simulink model "l8_ver118_07"  (Simulink 7.9) (continued)
== system l8_ver118_07/s1 == (continued)
      mask help:
      | Standard snap block, but with the following optional extras;
      |  1) Optionally delay the fabric trigger by a user-specified number of valids.
      |  2) Capture continuously after a trigger until a stop pulse is received. Data is captured into BRAM continuously, with old data being overwritten until a stop command is received. Then stop capturing when memory is filled. This enables you to get some data before and some data after the stop pulse. Use the 'tr_en_cnt' register to determine where you are in your vector. 'Addr' register's MSb now indicates 'busy capturing'.
      |  3) Optionally capture a value on an input port to a register at the same time as the first data sample.
      |  4) Implement counters using DSP48Es to save resources.
      mask init: same as l8_ver118_07/adcsnap0
      mask StyleString: popup(bram|dram),popup(1|2|3|4),popup(200|250|266|300|333),edit,popup(8|16|32|64|128),checkbox,checkbox,checkbox,checkbox
      mask CallbackString: snapshot_callback();||||||||
  p1im_firstcic : Subsystem  AncestorBlock=casper_library_scopes/snapshot  mask(snapshot){storage=bram, dram_dimm=1, dram_clock=200, nsamples=12, data_width=32, offset=off, circap=off, value=off, use_dsp48=on}
      mask prompts: storage "Storage medium"; dram_dimm "DRAM dimm"; dram_clock "DRAM clock rate"; nsamples "Number of Samples (2^?)"; data_width "Data width"; offset "Start delay support"; circap "Circular capture support"; value "Extra value capture support"; use_dsp48 "Use DSP48s to implement counters"
      mask help:
      | Standard snap block, but with the following optional extras;
      |  1) Optionally delay the fabric trigger by a user-specified number of valids.
      |  2) Capture continuously after a trigger until a stop pulse is received. Data is captured into BRAM continuously, with old data being overwritten until a stop command is received. Then stop capturing when memory is filled. This enables you to get some data before and some data after the stop pulse. Use the 'tr_en_cnt' register to determine where you are in your vector. 'Addr' register's MSb now indicates 'busy capturing'.
      |  3) Optionally capture a value on an input port to a register at the same time as the first data sample.
      |  4) Implement counters using DSP48Es to save resources.
      mask init: same as l8_ver118_07/adcsnap0
      mask StyleString: popup(bram|dram),popup(1|2|3|4),popup(200|250|266|300|333),edit,popup(8|16|32|64|128),checkbox,checkbox,checkbox,checkbox
      mask CallbackString: snapshot_callback();||||||||
  p1im_firstcic_full : Subsystem  AncestorBlock=casper_library_scopes/snapshot  mask(snapshot){storage=bram, dram_dimm=1, dram_clock=200, nsamples=12, data_width=64, offset=off, circap=off, value=off, use_dsp48=on}
      mask prompts: storage "Storage medium"; dram_dimm "DRAM dimm"; dram_clock "DRAM clock rate"; nsamples "Number of Samples (2^?)"; data_width "Data width"; offset "Start delay support"; circap "Circular capture support"; value "Extra value capture support"; use_dsp48 "Use DSP48s to implement counters"
      mask help:
      | Standard snap block, but with the following optional extras;
      |  1) Optionally delay the fabric trigger by a user-specified number of valids.
      |  2) Capture continuously after a trigger until a stop pulse is received. Data is captured into BRAM continuously, with old data being overwritten until a stop command is received. Then stop capturing when memory is filled. This enables you to get some data before and some data after the stop pulse. Use the 'tr_en_cnt' register to determine where you are in your vector. 'Addr' register's MSb now indicates 'busy capturing'.
      |  3) Optionally capture a value on an input port to a register at the same time as the first data sample.
      |  4) Implement counters using DSP48Es to save resources.
      mask init: same as l8_ver118_07/adcsnap0
      mask StyleString: popup(bram|dram),popup(1|2|3|4),popup(200|250|266|300|333),edit,popup(8|16|32|64|128),checkbox,checkbox,checkbox,checkbox
      mask CallbackString: snapshot_callback();||||||||
  p1im_halfband : Subsystem  AncestorBlock=casper_library_scopes/snapshot  mask(snapshot){storage=bram, dram_dimm=1, dram_clock=200, nsamples=12, data_width=32, offset=off, circap=off, value=off, use_dsp48=on}
      mask prompts: storage "Storage medium"; dram_dimm "DRAM dimm"; dram_clock "DRAM clock rate"; nsamples "Number of Samples (2^?)"; data_width "Data width"; offset "Start delay support"; circap "Circular capture support"; value "Extra value capture support"; use_dsp48 "Use DSP48s to implement counters"
      mask help:
      | Standard snap block, but with the following optional extras;
      |  1) Optionally delay the fabric trigger by a user-specified number of valids.
      |  2) Capture continuously after a trigger until a stop pulse is received. Data is captured into BRAM continuously, with old data being overwritten until a stop command is received. Then stop capturing when memory is filled. This enables you to get some data before and some data after the stop pulse. Use the 'tr_en_cnt' register to determine where you are in your vector. 'Addr' register's MSb now indicates 'busy capturing'.
      |  3) Optionally capture a value on an input port to a register at the same time as the first data sample.
      |  4) Implement counters using DSP48Es to save resources.
      mask init: same as l8_ver118_07/adcsnap0
      mask StyleString: popup(bram|dram),popup(1|2|3|4),popup(200|250|266|300|333),edit,popup(8|16|32|64|128),checkbox,checkbox,checkbox,checkbox
      mask CallbackString: snapshot_callback();||||||||
  p1re_dr16 : Subsystem  AncestorBlock=casper_library_scopes/snapshot  mask(snapshot){storage=bram, dram_dimm=1, dram_clock=200, nsamples=12, data_width=64, offset=off, circap=off, value=off, use_dsp48=on}
      mask prompts: storage "Storage medium"; dram_dimm "DRAM dimm"; dram_clock "DRAM clock rate"; nsamples "Number of Samples (2^?)"; data_width "Data width"; offset "Start delay support"; circap "Circular capture support"; value "Extra value capture support"; use_dsp48 "Use DSP48s to implement counters"
      mask help:
      | Standard snap block, but with the following optional extras;
      |  1) Optionally delay the fabric trigger by a user-specified number of valids.
      |  2) Capture continuously after a trigger until a stop pulse is received. Data is captured into BRAM continuously, with old data being overwritten until a stop command is received. Then stop capturing when memory is filled. This enables you to get some data before and some data after the stop pulse. Use the 'tr_en_cnt' register to determine where you are in your vector. 'Addr' register's MSb now indicates 'busy capturing'.
      |  3) Optionally capture a value on an input port to a register at the same time as the first data sample.
      |  4) Implement counters using DSP48Es to save resources.
      mask init: same as l8_ver118_07/adcsnap0
      mask StyleString: popup(bram|dram),popup(1|2|3|4),popup(200|250|266|300|333),edit,popup(8|16|32|64|128),checkbox,checkbox,checkbox,checkbox
      mask CallbackString: snapshot_callback();||||||||
  p1re_firstcic : Subsystem  AncestorBlock=casper_library_scopes/snapshot  mask(snapshot){storage=bram, dram_dimm=1, dram_clock=200, nsamples=12, data_width=32, offset=off, circap=off, value=off, use_dsp48=on}
      mask prompts: storage "Storage medium"; dram_dimm "DRAM dimm"; dram_clock "DRAM clock rate"; nsamples "Number of Samples (2^?)"; data_width "Data width"; offset "Start delay support"; circap "Circular capture support"; value "Extra value capture support"; use_dsp48 "Use DSP48s to implement counters"
      mask help:
      | Standard snap block, but with the following optional extras;
      |  1) Optionally delay the fabric trigger by a user-specified number of valids.
      |  2) Capture continuously after a trigger until a stop pulse is received. Data is captured into BRAM continuously, with old data being overwritten until a stop command is received. Then stop capturing when memory is filled. This enables you to get some data before and some data after the stop pulse. Use the 'tr_en_cnt' register to determine where you are in your vector. 'Addr' register's MSb now indicates 'busy capturing'.
      |  3) Optionally capture a value on an input port to a register at the same time as the first data sample.
      |  4) Implement counters using DSP48Es to save resources.
      mask init: same as l8_ver118_07/adcsnap0
      mask StyleString: popup(bram|dram),popup(1|2|3|4),popup(200|250|266|300|333),edit,popup(8|16|32|64|128),checkbox,checkbox,checkbox,checkbox
      mask CallbackString: snapshot_callback();||||||||
  p1re_firstcic_full : Subsystem  AncestorBlock=casper_library_scopes/snapshot  mask(snapshot){storage=bram, dram_dimm=1, dram_clock=200, nsamples=12, data_width=64, offset=off, circap=off, value=off, use_dsp48=on}
      mask prompts: storage "Storage medium"; dram_dimm "DRAM dimm"; dram_clock "DRAM clock rate"; nsamples "Number of Samples (2^?)"; data_width "Data width"; offset "Start delay support"; circap "Circular capture support"; value "Extra value capture support"; use_dsp48 "Use DSP48s to implement counters"
      mask help:
      | Standard snap block, but with the following optional extras;
      |  1) Optionally delay the fabric trigger by a user-specified number of valids.
      |  2) Capture continuously after a trigger until a stop pulse is received. Data is captured into BRAM continuously, with old data being overwritten until a stop command is received. Then stop capturing when memory is filled. This enables you to get some data before and some data after the stop pulse. Use the 'tr_en_cnt' register to determine where you are in your vector. 'Addr' register's MSb now indicates 'busy capturing'.
      |  3) Optionally capture a value on an input port to a register at the same time as the first data sample.
      |  4) Implement counters using DSP48Es to save resources.
      mask init: same as l8_ver118_07/adcsnap0
      mask StyleString: popup(bram|dram),popup(1|2|3|4),popup(200|250|266|300|333),edit,popup(8|16|32|64|128),checkbox,checkbox,checkbox,checkbox
      mask CallbackString: snapshot_callback();||||||||
  p1re_halfband : Subsystem  AncestorBlock=casper_library_scopes/snapshot  mask(snapshot){storage=bram, dram_dimm=1, dram_clock=200, nsamples=12, data_width=32, offset=off, circap=off, value=off, use_dsp48=on}
      mask prompts: storage "Storage medium"; dram_dimm "DRAM dimm"; dram_clock "DRAM clock rate"; nsamples "Number of Samples (2^?)"; data_width "Data width"; offset "Start delay support"; circap "Circular capture support"; value "Extra value capture support"; use_dsp48 "Use DSP48s to implement counters"
      mask help:
      | Standard snap block, but with the following optional extras;
      |  1) Optionally delay the fabric trigger by a user-specified number of valids.
      |  2) Capture continuously after a trigger until a stop pulse is received. Data is captured into BRAM continuously, with old data being overwritten until a stop command is received. Then stop capturing when memory is filled. This enables you to get some data before and some data after the stop pulse. Use the 'tr_en_cnt' register to determine where you are in your vector. 'Addr' register's MSb now indicates 'busy capturing'.
      |  3) Optionally capture a value on an input port to a register at the same time as the first data sample.
      |  4) Implement counters using DSP48Es to save resources.
      mask init: same as l8_ver118_07/adcsnap0
      mask StyleString: popup(bram|dram),popup(1|2|3|4),popup(200|250|266|300|333),edit,popup(8|16|32|64|128),checkbox,checkbox,checkbox,checkbox
      mask CallbackString: snapshot_callback();||||||||
  p2im_dr16 : Subsystem  AncestorBlock=casper_library_scopes/snapshot  mask(snapshot){storage=bram, dram_dimm=1, dram_clock=200, nsamples=12, data_width=64, offset=off, circap=off, value=off, use_dsp48=on}
      mask prompts: storage "Storage medium"; dram_dimm "DRAM dimm"; dram_clock "DRAM clock rate"; nsamples "Number of Samples (2^?)"; data_width "Data width"; offset "Start delay support"; circap "Circular capture support"; value "Extra value capture support"; use_dsp48 "Use DSP48s to implement counters"
      mask help:
      | Standard snap block, but with the following optional extras;
      |  1) Optionally delay the fabric trigger by a user-specified number of valids.
      |  2) Capture continuously after a trigger until a stop pulse is received. Data is captured into BRAM continuously, with old data being overwritten until a stop command is received. Then stop capturing when memory is filled. This enables you to get some data before and some data after the stop pulse. Use the 'tr_en_cnt' register to determine where you are in your vector. 'Addr' register's MSb now indicates 'busy capturing'.
      |  3) Optionally capture a value on an input port to a register at the same time as the first data sample.
      |  4) Implement counters using DSP48Es to save resources.
      mask init: same as l8_ver118_07/adcsnap0
      mask StyleString: popup(bram|dram),popup(1|2|3|4),popup(200|250|266|300|333),edit,popup(8|16|32|64|128),checkbox,checkbox,checkbox,checkbox
      mask CallbackString: snapshot_callback();||||||||
  p2im_firstcic : Subsystem  AncestorBlock=casper_library_scopes/snapshot  mask(snapshot){storage=bram, dram_dimm=1, dram_clock=200, nsamples=12, data_width=32, offset=off, circap=off, value=off, use_dsp48=on}
      mask prompts: storage "Storage medium"; dram_dimm "DRAM dimm"; dram_clock "DRAM clock rate"; nsamples "Number of Samples (2^?)"; data_width "Data width"; offset "Start delay support"; circap "Circular capture support"; value "Extra value capture support"; use_dsp48 "Use DSP48s to implement counters"
      mask help:
      | Standard snap block, but with the following optional extras;
      |  1) Optionally delay the fabric trigger by a user-specified number of valids.
      |  2) Capture continuously after a trigger until a stop pulse is received. Data is captured into BRAM continuously, with old data being overwritten until a stop command is received. Then stop capturing when memory is filled. This enables you to get some data before and some data after the stop pulse. Use the 'tr_en_cnt' register to determine where you are in your vector. 'Addr' register's MSb now indicates 'busy capturing'.
      |  3) Optionally capture a value on an input port to a register at the same time as the first data sample.
      |  4) Implement counters using DSP48Es to save resources.
      mask init: same as l8_ver118_07/adcsnap0
      mask StyleString: popup(bram|dram),popup(1|2|3|4),popup(200|250|266|300|333),edit,popup(8|16|32|64|128),checkbox,checkbox,checkbox,checkbox
      mask CallbackString: snapshot_callback();||||||||
  p2im_firstcic_full : Subsystem  AncestorBlock=casper_library_scopes/snapshot  mask(snapshot){storage=bram, dram_dimm=1, dram_clock=200, nsamples=12, data_width=64, offset=off, circap=off, value=off, use_dsp48=on}
      mask prompts: storage "Storage medium"; dram_dimm "DRAM dimm"; dram_clock "DRAM clock rate"; nsamples "Number of Samples (2^?)"; data_width "Data width"; offset "Start delay support"; circap "Circular capture support"; value "Extra value capture support"; use_dsp48 "Use DSP48s to implement counters"
      mask help:
      | Standard snap block, but with the following optional extras;
      |  1) Optionally delay the fabric trigger by a user-specified number of valids.
      |  2) Capture continuously after a trigger until a stop pulse is received. Data is captured into BRAM continuously, with old data being overwritten until a stop command is received. Then stop capturing when memory is filled. This enables you to get some data before and some data after the stop pulse. Use the 'tr_en_cnt' register to determine where you are in your vector. 'Addr' register's MSb now indicates 'busy capturing'.
      |  3) Optionally capture a value on an input port to a register at the same time as the first data sample.
      |  4) Implement counters using DSP48Es to save resources.
      mask init: same as l8_ver118_07/adcsnap0
      mask StyleString: popup(bram|dram),popup(1|2|3|4),popup(200|250|266|300|333),edit,popup(8|16|32|64|128),checkbox,checkbox,checkbox,checkbox
      mask CallbackString: snapshot_callback();||||||||
  p2im_halfband : Subsystem  AncestorBlock=casper_library_scopes/snapshot  mask(snapshot){storage=bram, dram_dimm=1, dram_clock=200, nsamples=12, data_width=32, offset=off, circap=off, value=off, use_dsp48=on}
      mask prompts: storage "Storage medium"; dram_dimm "DRAM dimm"; dram_clock "DRAM clock rate"; nsamples "Number of Samples (2^?)"; data_width "Data width"; offset "Start delay support"; circap "Circular capture support"; value "Extra value capture support"; use_dsp48 "Use DSP48s to implement counters"
      mask help:
      | Standard snap block, but with the following optional extras;
      |  1) Optionally delay the fabric trigger by a user-specified number of valids.
      |  2) Capture continuously after a trigger until a stop pulse is received. Data is captured into BRAM continuously, with old data being overwritten until a stop command is received. Then stop capturing when memory is filled. This enables you to get some data before and some data after the stop pulse. Use the 'tr_en_cnt' register to determine where you are in your vector. 'Addr' register's MSb now indicates 'busy capturing'.
      |  3) Optionally capture a value on an input port to a register at the same time as the first data sample.
      |  4) Implement counters using DSP48Es to save resources.
      mask init: same as l8_ver118_07/adcsnap0
      mask StyleString: popup(bram|dram),popup(1|2|3|4),popup(200|250|266|300|333),edit,popup(8|16|32|64|128),checkbox,checkbox,checkbox,checkbox
      mask CallbackString: snapshot_callback();||||||||
  p2re_dr16 : Subsystem  AncestorBlock=casper_library_scopes/snapshot  mask(snapshot){storage=bram, dram_dimm=1, dram_clock=200, nsamples=12, data_width=64, offset=off, circap=off, value=off, use_dsp48=on}
      mask prompts: storage "Storage medium"; dram_dimm "DRAM dimm"; dram_clock "DRAM clock rate"; nsamples "Number of Samples (2^?)"; data_width "Data width"; offset "Start delay support"; circap "Circular capture support"; value "Extra value capture support"; use_dsp48 "Use DSP48s to implement counters"
      mask help:
      | Standard snap block, but with the following optional extras;
      |  1) Optionally delay the fabric trigger by a user-specified number of valids.
      |  2) Capture continuously after a trigger until a stop pulse is received. Data is captured into BRAM continuously, with old data being overwritten until a stop command is received. Then stop capturing when memory is filled. This enables you to get some data before and some data after the stop pulse. Use the 'tr_en_cnt' register to determine where you are in your vector. 'Addr' register's MSb now indicates 'busy capturing'.
      |  3) Optionally capture a value on an input port to a register at the same time as the first data sample.
      |  4) Implement counters using DSP48Es to save resources.
      mask init: same as l8_ver118_07/adcsnap0
      mask StyleString: popup(bram|dram),popup(1|2|3|4),popup(200|250|266|300|333),edit,popup(8|16|32|64|128),checkbox,checkbox,checkbox,checkbox
      mask CallbackString: snapshot_callback();||||||||
  p2re_firstcic : Subsystem  AncestorBlock=casper_library_scopes/snapshot  mask(snapshot){storage=bram, dram_dimm=1, dram_clock=200, nsamples=12, data_width=32, offset=off, circap=off, value=off, use_dsp48=on}
      mask prompts: storage "Storage medium"; dram_dimm "DRAM dimm"; dram_clock "DRAM clock rate"; nsamples "Number of Samples (2^?)"; data_width "Data width"; offset "Start delay support"; circap "Circular capture support"; value "Extra value capture support"; use_dsp48 "Use DSP48s to implement counters"
      mask help:
      | Standard snap block, but with the following optional extras;
      |  1) Optionally delay the fabric trigger by a user-specified number of valids.
      |  2) Capture continuously after a trigger until a stop pulse is received. Data is captured into BRAM continuously, with old data being overwritten until a stop command is received. Then stop capturing when memory is filled. This enables you to get some data before and some data after the stop pulse. Use the 'tr_en_cnt' register to determine where you are in your vector. 'Addr' register's MSb now indicates 'busy capturing'.
      |  3) Optionally capture a value on an input port to a register at the same time as the first data sample.
      |  4) Implement counters using DSP48Es to save resources.
      mask init: same as l8_ver118_07/adcsnap0
      mask StyleString: popup(bram|dram),popup(1|2|3|4),popup(200|250|266|300|333),edit,popup(8|16|32|64|128),checkbox,checkbox,checkbox,checkbox
      mask CallbackString: snapshot_callback();||||||||
  p2re_firstcic_full : Subsystem  AncestorBlock=casper_library_scopes/snapshot  mask(snapshot){storage=bram, dram_dimm=1, dram_clock=200, nsamples=12, data_width=64, offset=off, circap=off, value=off, use_dsp48=on}
      mask prompts: storage "Storage medium"; dram_dimm "DRAM dimm"; dram_clock "DRAM clock rate"; nsamples "Number of Samples (2^?)"; data_width "Data width"; offset "Start delay support"; circap "Circular capture support"; value "Extra value capture support"; use_dsp48 "Use DSP48s to implement counters"
      mask help:
      | Standard snap block, but with the following optional extras;
      |  1) Optionally delay the fabric trigger by a user-specified number of valids.
      |  2) Capture continuously after a trigger until a stop pulse is received. Data is captured into BRAM continuously, with old data being overwritten until a stop command is received. Then stop capturing when memory is filled. This enables you to get some data before and some data after the stop pulse. Use the 'tr_en_cnt' register to determine where you are in your vector. 'Addr' register's MSb now indicates 'busy capturing'.
      |  3) Optionally capture a value on an input port to a register at the same time as the first data sample.
      |  4) Implement counters using DSP48Es to save resources.
      mask init: same as l8_ver118_07/adcsnap0
      mask StyleString: popup(bram|dram),popup(1|2|3|4),popup(200|250|266|300|333),edit,popup(8|16|32|64|128),checkbox,checkbox,checkbox,checkbox
      mask CallbackString: snapshot_callback();||||||||
  p2re_halfband : Subsystem  AncestorBlock=casper_library_scopes/snapshot  mask(snapshot){storage=bram, dram_dimm=1, dram_clock=200, nsamples=12, data_width=32, offset=off, circap=off, value=off, use_dsp48=on}
      mask prompts: storage "Storage medium"; dram_dimm "DRAM dimm"; dram_clock "DRAM clock rate"; nsamples "Number of Samples (2^?)"; data_width "Data width"; offset "Start delay support"; circap "Circular capture support"; value "Extra value capture support"; use_dsp48 "Use DSP48s to implement counters"
      mask help:
      | Standard snap block, but with the following optional extras;
      |  1) Optionally delay the fabric trigger by a user-specified number of valids.
      |  2) Capture continuously after a trigger until a stop pulse is received. Data is captured into BRAM continuously, with old data being overwritten until a stop command is received. Then stop capturing when memory is filled. This enables you to get some data before and some data after the stop pulse. Use the 'tr_en_cnt' register to determine where you are in your vector. 'Addr' register's MSb now indicates 'busy capturing'.
      |  3) Optionally capture a value on an input port to a register at the same time as the first data sample.
      |  4) Implement counters using DSP48Es to save resources.
      mask init: same as l8_ver118_07/adcsnap0
      mask StyleString: popup(bram|dram),popup(1|2|3|4),popup(200|250|266|300|333),edit,popup(8|16|32|64|128),checkbox,checkbox,checkbox,checkbox
      mask CallbackString: snapshot_callback();||||||||
  quant : Subsystem
  pol1_re : Outport
  pol1_im : Outport  Port=2
  pol2_re : Outport  Port=3
  pol2_im : Outport  Port=4
  sync : Outport  Port=5
  wires:
  pol1_in0/1 -> [pol1_0]
  pol1_in1/1 -> [pol1_1]
  pol1_in2/1 -> [pol1_2]
  pol1_in3/1 -> [pol1_3]
  pol1_in4/1 -> [pol1_4]
  pol1_in5/1 -> [pol1_5]
  pol1_in6/1 -> [pol1_6]
  pol1_in7/1 -> [pol1_7]
  pol2_in0/1 -> [pol2_0]
  pol2_in1/1 -> [pol2_1]
  pol2_in2/1 -> [pol2_2]
  pol2_in3/1 -> [pol2_3]
  pol2_in4/1 -> [pol2_4]
  pol2_in5/1 -> [pol2_5]
  pol2_in6/1 -> [pol2_6]
  pol2_in7/1 -> [pol2_7]
  [mixer_addr] -> lo_2/1(addr)
  [mixer_addr] -> lo_4/1(addr)
  [mixer_addr] -> lo_6/1(addr)
  lo_8/1(pol1_im) -> [pol1_imag_8]
  lo_8/2(pol2_im) -> [pol2_imag_8]
  lo_8/3(pol1_re) -> [pol1_real_8]
  lo_8/4(pol2_re) -> [pol2_real_8]
  [mixer_addr] -> lo_8/1(addr)
  [pol2_8] -> lo_8/3(pol2)
  [pol1_8] -> lo_8/2(pol1)
  [mixer_addr] -> lo_0/1(addr)
  [pol1_0] -> lo_0/2(pol1)
  [pol2_0] -> lo_0/3(pol2)
  lo_0/1(pol1_im) -> [pol1_imag_0]
  lo_0/2(pol2_im) -> [pol2_imag_0]
  lo_0/3(pol1_re) -> [pol1_real_0]
  lo_0/4(pol2_re) -> [pol2_real_0]
  [pol1_2] -> lo_2/2(pol1)
  [pol2_2] -> lo_2/3(pol2)
  [pol1_4] -> lo_4/2(pol1)
  [pol2_4] -> lo_4/3(pol2)
  [pol1_6] -> lo_6/2(pol1)
  [pol2_6] -> lo_6/3(pol2)
  lo_6/1(pol1_im) -> [pol1_imag_6]
  lo_6/2(pol2_im) -> [pol2_imag_6]
  lo_6/3(pol1_re) -> [pol1_real_6]
  lo_6/4(pol2_re) -> [pol2_real_6]
  [mixer_addr] -> lo_10/1(addr)
  [pol1_10] -> lo_10/2(pol1)
  [pol2_10] -> lo_10/3(pol2)
  lo_10/1(pol1_im) -> [pol1_imag_10]
  lo_10/2(pol2_im) -> [pol2_imag_10]
  lo_10/3(pol1_re) -> [pol1_real_10]
  lo_10/4(pol2_re) -> [pol2_real_10]
  [mixer_addr] -> lo_12/1(addr)
  [pol1_12] -> lo_12/2(pol1)
  [pol2_12] -> lo_12/3(pol2)
  lo_12/1(pol1_im) -> [pol1_imag_12]
  lo_12/2(pol2_im) -> [pol2_imag_12]
  lo_12/3(pol1_re) -> [pol1_real_12]
  lo_12/4(pol2_re) -> [pol2_real_12]
  [mixer_addr] -> lo_14/1(addr)
  [pol1_14] -> lo_14/2(pol1)
  [pol2_14] -> lo_14/3(pol2)
  lo_14/1(pol1_im) -> [pol1_imag_14]
  lo_14/2(pol2_im) -> [pol2_imag_14]
  lo_14/3(pol1_re) -> [pol1_real_14]
  lo_14/4(pol2_re) -> [pol2_real_14]
  lo_4/1(pol1_im) -> [pol1_imag_4]
  lo_4/2(pol2_im) -> [pol2_imag_4]
  lo_4/3(pol1_re) -> [pol1_real_4]
  lo_4/4(pol2_re) -> [pol2_real_4]
  adc_CIC_sync/1 -> [cic_sync]
  [pol1_real_quant] -> pol1_re/1
  cic_stage_dec2/2(sync_out) -> cic_stage_dec1/2(sync_in)
  cic_stage_dec2/1(out) -> cic_stage_dec1/1(in)
  Halfband0/1(out) -> Convert1/1
  [cic_sync] -> cic_stage_dec2/2(sync_in)
  Convert2/1 -> Halfband0/1(in), [pol1_real_1stcic]
  Convert1/1 -> cic_stage_dec2/1(in), [pol1_real_halfband]
  Convert3/1 -> [pol1_real]
  [pol1_imag_quant] -> pol1_im/1
  quant/1(Out1) -> [pol1_real_quant]
  quant/2(Out2) -> [pol1_imag_quant]
  [pol1_real] -> quant/1(In2)
  [pol1_imag] -> quant/2(In3)
  [pol2_real] -> quant/3(In4)
  [pol2_imag] -> quant/4(In5)
  quant/3(Out3) -> [pol2_real_quant]
  quant/4(Out4) -> [pol2_imag_quant]
  [pol2_real_quant] -> pol2_re/1
  [pol2_imag_quant] -> pol2_im/1
  pol1_in8/1 -> [pol1_8]
  pol1_in9/1 -> [pol1_9]
  pol1_in10/1 -> [pol1_10]
  pol1_in11/1 -> [pol1_11]
  pol1_in12/1 -> [pol1_12]
  pol1_in13/1 -> [pol1_13]
  pol1_in14/1 -> [pol1_14]
  pol1_in15/1 -> [pol1_15]
  pol2_in8/1 -> [pol2_8]
  pol2_in9/1 -> [pol2_9]
  pol2_in10/1 -> [pol2_10]
  pol2_in11/1 -> [pol2_11]
  pol2_in12/1 -> [pol2_12]
  pol2_in13/1 -> [pol2_13]
  pol2_in14/1 -> [pol2_14]
  pol2_in15/1 -> [pol2_15]
  [mixer_addr] -> lo_1/1(addr)
  [pol1_1] -> lo_1/2(pol1)
  [pol2_1] -> lo_1/3(pol2)
  lo_1/1(pol1_im) -> [pol1_imag_1]
  lo_1/2(pol2_im) -> [pol2_imag_1]
  lo_1/3(pol1_re) -> [pol1_real_1]
  lo_1/4(pol2_re) -> [pol2_real_1]
  [mixer_addr] -> lo_9/1(addr)
  [pol1_9] -> lo_9/2(pol1)
  [pol2_9] -> lo_9/3(pol2)
  lo_9/1(pol1_im) -> [pol1_imag_9]
  lo_9/2(pol2_im) -> [pol2_imag_9]
  lo_9/3(pol1_re) -> [pol1_real_9]
  lo_9/4(pol2_re) -> [pol2_real_9]
  [mixer_addr] -> lo_3/1(addr)
  [pol1_3] -> lo_3/2(pol1)
  [pol2_3] -> lo_3/3(pol2)
  [mixer_addr] -> lo_11/1(addr)
  [pol1_11] -> lo_11/2(pol1)
  [pol2_11] -> lo_11/3(pol2)
  lo_11/1(pol1_im) -> [pol1_imag_11]
  lo_11/2(pol2_im) -> [pol2_imag_11]
  lo_11/3(pol1_re) -> [pol1_real_11]
  lo_11/4(pol2_re) -> [pol2_real_11]
  [mixer_addr] -> lo_5/1(addr)
  [pol1_5] -> lo_5/2(pol1)
  [pol2_5] -> lo_5/3(pol2)
  lo_5/1(pol1_im) -> [pol1_imag_5]
  lo_5/2(pol2_im) -> [pol2_imag_5]
  lo_5/3(pol1_re) -> [pol1_real_5]
  lo_5/4(pol2_re) -> [pol2_real_5]
  [mixer_addr] -> lo_13/1(addr)
  [pol1_13] -> lo_13/2(pol1)
  [pol2_13] -> lo_13/3(pol2)
  lo_13/1(pol1_im) -> [pol1_imag_13]
  lo_13/2(pol2_im) -> [pol2_imag_13]
  lo_13/3(pol1_re) -> [pol1_real_13]
  lo_13/4(pol2_re) -> [pol2_real_13]
  [mixer_addr] -> lo_7/1(addr)
  [pol1_7] -> lo_7/2(pol1)
  [pol2_7] -> lo_7/3(pol2)
  lo_7/1(pol1_im) -> [pol1_imag_7]
  lo_7/2(pol2_im) -> [pol2_imag_7]
  lo_7/3(pol1_re) -> [pol1_real_7]
  lo_7/4(pol2_re) -> [pol2_real_7]
  [mixer_addr] -> lo_15/1(addr)
  [pol1_15] -> lo_15/2(pol1)
  [pol2_15] -> lo_15/3(pol2)
  lo_15/1(pol1_im) -> [pol1_imag_15]
  lo_15/2(pol2_im) -> [pol2_imag_15]
  lo_15/3(pol1_re) -> [pol1_real_15]
  lo_15/4(pol2_re) -> [pol2_real_15]
  lo_2/2(pol2_im) -> [pol2_imag_2]
  lo_2/1(pol1_im) -> [pol1_imag_2]
  lo_2/3(pol1_re) -> [pol1_real_2]
  lo_2/4(pol2_re) -> [pol2_real_2]
  lo_3/1(pol1_im) -> [pol1_imag_3]
  lo_3/2(pol2_im) -> [pol2_imag_3]
  lo_3/3(pol1_re) -> [pol1_real_3]
  lo_3/4(pol2_re) -> [pol2_real_3]
  [cic_sync] -> cic_pow2_0/17(sync_in)
  [pol1_real_0] -> cic_pow2_0/1(in1)
  [pol1_real_1] -> cic_pow2_0/2(in2)
  [pol1_real_2] -> cic_pow2_0/3(in3)
  [pol1_real_3] -> cic_pow2_0/4(in4)
  [pol1_real_4] -> cic_pow2_0/5(in5)
  [pol1_real_5] -> cic_pow2_0/6(in6)
  [pol1_real_6] -> cic_pow2_0/7(in7)
  [pol1_real_7] -> cic_pow2_0/8(in8)
  [pol1_real_8] -> cic_pow2_0/9(in9)
  [pol1_real_9] -> cic_pow2_0/10(in10)
  [pol1_real_10] -> cic_pow2_0/11(in11)
  [pol1_real_11] -> cic_pow2_0/12(in12)
  [pol1_real_12] -> cic_pow2_0/13(in13)
  [pol1_real_13] -> cic_pow2_0/14(in14)
  [pol1_real_14] -> cic_pow2_0/15(in15)
  [pol1_real_15] -> cic_pow2_0/16(in16)
  cic_stage_dec1/1(out) -> Convert3/1
  cic_stage_dec1/2(sync_out) -> sync/1
  Constant19/1 -> p1re_halfband/3(trig), p1re_halfband/2(we)
  [pol1_real_halfband] -> p1re_halfband/1(din)
  Constant1/1 -> p1re_firstcic/3(trig), p1re_firstcic/2(we)
  [pol1_real_1stcic] -> p1re_firstcic/1(din)
  Reinterpret1/1 -> Convert2/1
  Constant2/1 -> mixer_p2im/2(we), mixer_p2im/3(trig)
  bus_create/1(bus_out) -> mixer_p2im/1(din)
  Slice/1 -> bus_create/1(in1)
  Slice1/1 -> bus_create/2(in2)
  Slice2/1 -> bus_create/3(in3)
  Slice3/1 -> bus_create/4(in4)
  Slice4/1 -> bus_create/5(in5)
  Slice5/1 -> bus_create/6(in6)
  Slice6/1 -> bus_create/7(in7)
  Slice7/1 -> bus_create/8(in8)
  Slice8/1 -> bus_create/9(in9)
  Slice9/1 -> bus_create/10(in10)
  Slice10/1 -> bus_create/11(in11)
  Slice11/1 -> bus_create/12(in12)
  Slice12/1 -> bus_create/13(in13)
  Slice13/1 -> bus_create/14(in14)
  Slice14/1 -> bus_create/15(in15)
  Slice15/1 -> bus_create/16(in16)
  Constant3/1 -> p1re_firstcic_full/3(trig), p1re_firstcic_full/2(we)
  [pol1_re_1stcic_full] -> Reinterpret5/1
  Reinterpret5/1 -> Convert12/1
  Convert12/1 -> p1re_firstcic_full/1(din)
  cic_stage_dec9/1(out) -> Reinterpret1/1, [pol1_re_1stcic_full]
  cic_stage_dec9/2(sync_out) -> Halfband0/2(sync)
  Constant4/1 -> p1re_dr16/3(trig), p1re_dr16/2(we)
  [pol1_re_16dr] -> Reinterpret6/1
  Reinterpret6/1 -> Convert13/1
  Convert13/1 -> p1re_dr16/1(din)
  mixer_addr/1 -> Relational/1, Delay80/1
  Delay80/1 -> [mixer_addr]
  Relational/1 -> mixer_addr/1
  mixer_cnt/1 -> Relational/2
  Constant15/1 -> mixer_cnt/1
  [pol1_imag_0] -> cic_pow2_1/1(in1)
  [pol1_imag_1] -> cic_pow2_1/2(in2)
  [pol1_imag_2] -> cic_pow2_1/3(in3)
  [pol1_imag_3] -> cic_pow2_1/4(in4)
  [pol1_imag_4] -> cic_pow2_1/5(in5)
  [pol1_imag_5] -> cic_pow2_1/6(in6)
  [pol1_imag_6] -> cic_pow2_1/7(in7)
  [pol1_imag_7] -> cic_pow2_1/8(in8)
  [pol1_imag_8] -> cic_pow2_1/9(in9)
  [pol1_imag_9] -> cic_pow2_1/10(in10)
  [pol1_imag_10] -> cic_pow2_1/11(in11)
  [pol1_imag_11] -> cic_pow2_1/12(in12)
  [pol1_imag_12] -> cic_pow2_1/13(in13)
  [pol1_imag_13] -> cic_pow2_1/14(in14)
  [pol1_imag_14] -> cic_pow2_1/15(in15)
  [pol1_imag_15] -> cic_pow2_1/16(in16)
  [cic_sync] -> cic_pow2_1/17(sync_in)
  cic_pow2_0/1(out1) -> cic_stage_dec9/1(in), [pol1_re_16dr]
  cic_pow2_0/2(sync_out) -> cic_stage_dec9/2(sync_in)
  cic_stage_dec4/2(sync_out) -> cic_stage_dec3/2(sync_in)
  cic_stage_dec4/1(out) -> cic_stage_dec3/1(in)
  Halfband1/1(out) -> Convert4/1
  [cic_sync] -> cic_stage_dec4/2(sync_in)
  Convert5/1 -> Halfband1/1(in), [pol1_imag_1stcic]
  Convert4/1 -> cic_stage_dec4/1(in), [pol1_imag_halfband]
  Convert6/1 -> [pol1_imag]
  cic_stage_dec3/1(out) -> Convert6/1
  Reinterpret2/1 -> Convert5/1
  cic_stage_dec5/1(out) -> Reinterpret2/1, [pol1_im_1stcic_full]
  cic_stage_dec5/2(sync_out) -> Halfband1/2(sync)
  cic_pow2_1/1(out1) -> cic_stage_dec5/1(in), [pol1_im_16dr]
  cic_pow2_1/2(sync_out) -> cic_stage_dec5/2(sync_in)
  Constant6/1 -> p1im_halfband/3(trig), p1im_halfband/2(we)
  [pol1_imag_halfband] -> p1im_halfband/1(din)
  Constant5/1 -> p1im_firstcic/3(trig), p1im_firstcic/2(we)
  [pol1_imag_1stcic] -> p1im_firstcic/1(din)
  Constant7/1 -> p1im_firstcic_full/3(trig), p1im_firstcic_full/2(we)
  [pol1_im_1stcic_full] -> Reinterpret3/1
  Reinterpret3/1 -> Convert7/1
  Convert7/1 -> p1im_firstcic_full/1(din)
  Constant8/1 -> p1im_dr16/3(trig), p1im_dr16/2(we)
  [pol1_im_16dr] -> Reinterpret4/1
  Reinterpret4/1 -> Convert8/1
  Convert8/1 -> p1im_dr16/1(din)
  [pol1_real_0] -> Gateway Out4/1
  [pol1_imag_0] -> Gateway Out1/1
  [pol2_real_0] -> Gateway Out2/1
  [pol2_imag_0] -> Gateway Out3/1
  Gateway Out4/1 -> Scope/1
  Gateway Out1/1 -> Scope/2
  Gateway Out2/1 -> Scope/3
  Gateway Out3/1 -> Scope/4
  cic_stage_dec7/2(sync_out) -> cic_stage_dec6/2(sync_in)
  cic_stage_dec7/1(out) -> cic_stage_dec6/1(in)
  Halfband2/1(out) -> Convert9/1
  [cic_sync] -> cic_stage_dec7/2(sync_in)
  Convert10/1 -> Halfband2/1(in), [pol2_real_1stcic]
  Convert9/1 -> cic_stage_dec7/1(in), [pol2_real_halfband]
  Convert11/1 -> [pol2_real]
  cic_stage_dec6/1(out) -> Convert11/1
  Reinterpret7/1 -> Convert10/1
  cic_stage_dec8/1(out) -> Reinterpret7/1, [pol2_re_1stcic_full]
  cic_stage_dec8/2(sync_out) -> Halfband2/2(sync)
  cic_pow2_2/1(out1) -> cic_stage_dec8/1(in), [pol2_re_16dr]
  cic_pow2_2/2(sync_out) -> cic_stage_dec8/2(sync_in)
  [pol2_real_0] -> cic_pow2_2/1(in1)
  [pol2_real_1] -> cic_pow2_2/2(in2)
  [pol2_real_2] -> cic_pow2_2/3(in3)
  [pol2_real_3] -> cic_pow2_2/4(in4)
  [pol2_real_4] -> cic_pow2_2/5(in5)
  [pol2_real_5] -> cic_pow2_2/6(in6)
  [pol2_real_6] -> cic_pow2_2/7(in7)
  [pol2_real_7] -> cic_pow2_2/8(in8)
  [pol2_real_8] -> cic_pow2_2/9(in9)
  [pol2_real_9] -> cic_pow2_2/10(in10)
  [pol2_real_10] -> cic_pow2_2/11(in11)
  [pol2_real_11] -> cic_pow2_2/12(in12)
  [pol2_real_12] -> cic_pow2_2/13(in13)
  [pol2_real_13] -> cic_pow2_2/14(in14)
  [pol2_real_14] -> cic_pow2_2/15(in15)
  [pol2_real_15] -> cic_pow2_2/16(in16)
  [cic_sync] -> cic_pow2_2/17(sync_in)
  Constant10/1 -> p2re_halfband/3(trig), p2re_halfband/2(we)
  [pol2_real_halfband] -> p2re_halfband/1(din)
  Constant9/1 -> p2re_firstcic/3(trig), p2re_firstcic/2(we)
  [pol2_real_1stcic] -> p2re_firstcic/1(din)
  Constant11/1 -> p2re_firstcic_full/3(trig), p2re_firstcic_full/2(we)
  [pol2_re_1stcic_full] -> Reinterpret8/1
  Reinterpret8/1 -> Convert14/1
  Convert14/1 -> p2re_firstcic_full/1(din)
  Constant12/1 -> p2re_dr16/3(trig), p2re_dr16/2(we)
  [pol2_re_16dr] -> Reinterpret9/1
  Reinterpret9/1 -> Convert15/1
  Convert15/1 -> p2re_dr16/1(din)
  cic_stage_dec11/2(sync_out) -> cic_stage_dec10/2(sync_in)
  cic_stage_dec11/1(out) -> cic_stage_dec10/1(in)
  Halfband3/1(out) -> Convert16/1
  [cic_sync] -> cic_stage_dec11/2(sync_in)
  Convert17/1 -> Halfband3/1(in), [pol2_imag_1stcic]
  Convert16/1 -> cic_stage_dec11/1(in), [pol2_imag_halfband]
  Convert18/1 -> [pol2_imag]
  cic_stage_dec10/1(out) -> Convert18/1
  Reinterpret10/1 -> Convert17/1
  cic_stage_dec12/1(out) -> Reinterpret10/1, [pol2_im_1stcic_full]
  cic_stage_dec12/2(sync_out) -> Halfband3/2(sync)
  cic_pow2_3/1(out1) -> cic_stage_dec12/1(in), [pol2_im_16dr]
  cic_pow2_3/2(sync_out) -> cic_stage_dec12/2(sync_in)
  [pol2_imag_0] -> cic_pow2_3/1(in1)
  [pol2_imag_1] -> cic_pow2_3/2(in2)
  [pol2_imag_2] -> cic_pow2_3/3(in3)
  [pol2_imag_3] -> cic_pow2_3/4(in4)
  [pol2_imag_4] -> cic_pow2_3/5(in5)
  [pol2_imag_5] -> cic_pow2_3/6(in6)
  [pol2_imag_6] -> cic_pow2_3/7(in7)
  [pol2_imag_7] -> cic_pow2_3/8(in8)
  [pol2_imag_8] -> cic_pow2_3/9(in9)
  [pol2_imag_9] -> cic_pow2_3/10(in10)
  [pol2_imag_10] -> cic_pow2_3/11(in11)
  [pol2_imag_11] -> cic_pow2_3/12(in12)
  [pol2_imag_12] -> cic_pow2_3/13(in13)
  [pol2_imag_13] -> cic_pow2_3/14(in14)
  [pol2_imag_14] -> cic_pow2_3/15(in15)
  [pol2_imag_15] -> cic_pow2_3/16(in16)
  [cic_sync] -> cic_pow2_3/17(sync_in)
  Constant14/1 -> p2im_halfband/3(trig), p2im_halfband/2(we)
  [pol2_imag_halfband] -> p2im_halfband/1(din)
  Constant13/1 -> p2im_firstcic/3(trig), p2im_firstcic/2(we)
  [pol2_imag_1stcic] -> p2im_firstcic/1(din)
  Constant16/1 -> p2im_firstcic_full/3(trig), p2im_firstcic_full/2(we)
  [pol2_im_1stcic_full] -> Reinterpret11/1
  Reinterpret11/1 -> Convert19/1
  Convert19/1 -> p2im_firstcic_full/1(din)
  Constant17/1 -> p2im_dr16/3(trig), p2im_dr16/2(we)
  [pol2_im_16dr] -> Reinterpret12/1
  Reinterpret12/1 -> Convert20/1
  Convert20/1 -> p2im_dr16/1(din)
  Constant18/1 -> mixer_p1re/2(we), mixer_p1re/3(trig)
  bus_create1/1(bus_out) -> mixer_p1re/1(din)
  [pol1_real_0] -> Slice16/1
  [pol1_real_1] -> Slice17/1
  [pol1_real_2] -> Slice24/1
  [pol1_real_3] -> Slice25/1
  [pol1_real_4] -> Slice26/1
  [pol1_real_5] -> Slice27/1
  [pol1_real_6] -> Slice28/1
  [pol1_real_7] -> Slice29/1
  [pol1_real_8] -> Slice30/1
  [pol1_real_9] -> Slice31/1
  [pol1_real_10] -> Slice18/1
  [pol1_real_11] -> Slice19/1
  [pol1_real_12] -> Slice20/1
  [pol1_real_13] -> Slice21/1
  [pol1_real_14] -> Slice22/1
  [pol1_real_15] -> Slice23/1
  Slice16/1 -> bus_create1/1(in1)
  Slice17/1 -> bus_create1/2(in2)
  Slice24/1 -> bus_create1/3(in3)
  Slice25/1 -> bus_create1/4(in4)
  Slice26/1 -> bus_create1/5(in5)
  Slice27/1 -> bus_create1/6(in6)
  Slice28/1 -> bus_create1/7(in7)
  Slice29/1 -> bus_create1/8(in8)
  Slice30/1 -> bus_create1/9(in9)
  Slice31/1 -> bus_create1/10(in10)
  Slice18/1 -> bus_create1/11(in11)
  Slice19/1 -> bus_create1/12(in12)
  Slice20/1 -> bus_create1/13(in13)
  Slice21/1 -> bus_create1/14(in14)
  Slice22/1 -> bus_create1/15(in15)
  Slice23/1 -> bus_create1/16(in16)
  Constant20/1 -> mixer_p1im/2(we), mixer_p1im/3(trig)
  bus_create2/1(bus_out) -> mixer_p1im/1(din)
  Slice32/1 -> bus_create2/1(in1)
  Slice33/1 -> bus_create2/2(in2)
  Slice40/1 -> bus_create2/3(in3)
  Slice41/1 -> bus_create2/4(in4)
  Slice42/1 -> bus_create2/5(in5)
  Slice43/1 -> bus_create2/6(in6)
  Slice44/1 -> bus_create2/7(in7)
  Slice45/1 -> bus_create2/8(in8)
  Slice46/1 -> bus_create2/9(in9)
  Slice47/1 -> bus_create2/10(in10)
  Slice34/1 -> bus_create2/11(in11)
  Slice35/1 -> bus_create2/12(in12)
  Slice36/1 -> bus_create2/13(in13)
  Slice37/1 -> bus_create2/14(in14)
  Slice38/1 -> bus_create2/15(in15)
  Slice39/1 -> bus_create2/16(in16)
  Constant21/1 -> mixer_p2re/2(we), mixer_p2re/3(trig)
  bus_create3/1(bus_out) -> mixer_p2re/1(din)
  Slice48/1 -> bus_create3/1(in1)
  Slice49/1 -> bus_create3/2(in2)
  Slice56/1 -> bus_create3/3(in3)
  Slice57/1 -> bus_create3/4(in4)
  Slice58/1 -> bus_create3/5(in5)
  Slice59/1 -> bus_create3/6(in6)
  Slice60/1 -> bus_create3/7(in7)
  Slice61/1 -> bus_create3/8(in8)
  Slice62/1 -> bus_create3/9(in9)
  Slice63/1 -> bus_create3/10(in10)
  Slice50/1 -> bus_create3/11(in11)
  Slice51/1 -> bus_create3/12(in12)
  Slice52/1 -> bus_create3/13(in13)
  Slice53/1 -> bus_create3/14(in14)
  Slice54/1 -> bus_create3/15(in15)
  Slice55/1 -> bus_create3/16(in16)
  [pol2_imag_0] -> Slice/1
  [pol2_imag_1] -> Slice1/1
  [pol2_imag_2] -> Slice2/1
  [pol2_imag_3] -> Slice3/1
  [pol2_imag_4] -> Slice4/1
  [pol2_imag_5] -> Slice5/1
  [pol2_imag_6] -> Slice6/1
  [pol2_imag_7] -> Slice7/1
  [pol2_imag_8] -> Slice8/1
  [pol2_imag_9] -> Slice9/1
  [pol2_imag_10] -> Slice10/1
  [pol2_imag_11] -> Slice11/1
  [pol2_imag_12] -> Slice12/1
  [pol2_imag_13] -> Slice13/1
  [pol2_imag_14] -> Slice14/1
  [pol2_imag_15] -> Slice15/1
  [pol2_real_0] -> Slice48/1
  [pol2_real_1] -> Slice49/1
  [pol2_real_2] -> Slice56/1
  [pol2_real_3] -> Slice57/1
  [pol2_real_4] -> Slice58/1
  [pol2_real_5] -> Slice59/1
  [pol2_real_6] -> Slice60/1
  [pol2_real_7] -> Slice61/1
  [pol2_real_8] -> Slice62/1
  [pol2_real_9] -> Slice63/1
  [pol2_real_10] -> Slice50/1
  [pol2_real_11] -> Slice51/1
  [pol2_real_12] -> Slice52/1
  [pol2_real_13] -> Slice53/1
  [pol2_real_14] -> Slice54/1
  [pol2_real_15] -> Slice55/1
  [pol1_imag_0] -> Slice32/1
  [pol1_imag_1] -> Slice33/1
  [pol1_imag_2] -> Slice40/1
  [pol1_imag_3] -> Slice41/1
  [pol1_imag_4] -> Slice42/1
  [pol1_imag_5] -> Slice43/1
  [pol1_imag_6] -> Slice44/1
  [pol1_imag_7] -> Slice45/1
  [pol1_imag_8] -> Slice46/1
  [pol1_imag_9] -> Slice47/1
  [pol1_imag_10] -> Slice34/1
  [pol1_imag_11] -> Slice35/1
  [pol1_imag_12] -> Slice36/1
  [pol1_imag_13] -> Slice37/1
  [pol1_imag_14] -> Slice38/1
  [pol1_imag_15] -> Slice39/1
